simulink model "wlan_phy_rx_pmd"  (Simulink 7.8)
settings: Solver=VariableStepDiscrete  SolverMode=SingleTasking  StartTime=0.0  StopTime=simtime  RelTol=1e-3
PostLoadFcn:
  | wlan_phy_rx_init
InitFcn:
  | wlan_phy_rx_init
StartFcn:
  | wlan_phy_rx_init
values from neighbouring files (symbols this model uses):
  barker_seq20 = 0.95 * circshift(barker_seq20, [0 4]) ./ max(abs(barker_seq20))    [wlan_phy_rx_init.m]
  bit_scrambler_lfsr_bytes = bi2de(reshape(repmat(scr, 1, 8), 8, 127)', 'left-msb')    [wlan_phy_rx_init.m]
  deinterleave_ROM = [deinterleave_ROM interleave_64QAM zeros(1, 512-length(interleave_64QAM))]    [wlan_phy_rx_init.m]
  longCorr_coef_i = [3*real(LTS_corr)]    [wlan_phy_rx_init.m]
  longCorr_coef_q = [3*imag(LTS_corr)]    [wlan_phy_rx_init.m]
  MAX_CP_LEN = 32    [wlan_phy_rx_init.m]
  MAX_NCBPS = 288    [wlan_phy_rx_init.m]
  MAX_NUM_BYTES = 4096    [wlan_phy_rx_init.m]
  MAX_NUM_SC = 64    [wlan_phy_rx_init.m]
  MAX_NUM_SYMS = 600    [wlan_phy_rx_init.m]
  n_bits_preFFT_sampBuff = ceil(log2(4*MAX_NUM_SC))    [wlan_phy_rx_init.m]
  NCBPS_16QAM = 192    [wlan_phy_rx_init.m]
  NCBPS_64QAM = 288    [wlan_phy_rx_init.m]
  NCBPS_BPSK = 48    [wlan_phy_rx_init.m]
  NCBPS_QPSK = 96    [wlan_phy_rx_init.m]
  NDBPS_16QAM12 = 96    [wlan_phy_rx_init.m]
  NDBPS_16QAM34 = 144    [wlan_phy_rx_init.m]
  NDBPS_64QAM23 = 192    [wlan_phy_rx_init.m]
  NDBPS_64QAM34 = 216    [wlan_phy_rx_init.m]
  NDBPS_BPSK12 = 24    [wlan_phy_rx_init.m]
  NDBPS_BPSK34 = 36    [wlan_phy_rx_init.m]
  NDBPS_QPSK12 = 48    [wlan_phy_rx_init.m]
  NDBPS_QPSK34 = 72    [wlan_phy_rx_init.m]
  paylod_vec_samp_time = 8    [wlan_phy_rx_init.m]
  PHY_MIN_PKT_LEN = 14    [wlan_phy_rx_init.m]
  scr_ind_rev = zeros(1,128)    [wlan_phy_rx_init.m]
undefined symbols (not defined in the file or in its folder): gcbh, xlBlockLoadCallback, xlGetNormalizedPeriod, xlipgetpartsetting
libraries: xbsIndex_r4 x3119, simulink x1, xbsEDKLib_r4 x1
(part 3 of 19)

== system wlan_phy_rx_pmd/FCS & Pkt Buf ==
  DSSS data : Inport
  OFDM data : Inport  Port=2
  Bus Selector : BusSelector  OutputSignals=byte_valid,byte_index,byte,last_byte
  Bus Selector1 : BusSelector  OutputSignals=byte_valid,byte_index,byte,last_byte
  Bytes In : Scope  NumInputPorts=8
      settings: TimeRange=10000  YMin=0~0~0~-1~-1~-1~-1~-1  YMax=1~90~225~1~1~1~1~1  SaveName=ScopeData36
  FCS Calc : Subsystem
  Gateway Out{1..4,8..9,11..12} (8 alike) : xbsIndex_r4/Gateway Out  inherit_from_input=off  hdl_port=off  timing_constraint=None  locs_specified=off  LOCs={}  UseAsDAC=off  DACChannel='1'  block_type=gatewayout
  Pkt Buf Interface : Subsystem
  wires:
  OFDM data/1 -> Bus Selector/1, Pkt Buf Interface/2(OFDM data), FCS Calc/2(OFDM data)
  DSSS data/1 -> Bus Selector1/1, Pkt Buf Interface/1(DSSS data), FCS Calc/1(DSSS data)
  Bus Selector1/1 -> Gateway Out9/1  "<byte_valid>"
  Bus Selector1/2 -> Gateway Out8/1  "<byte_index>"
  Bus Selector1/3 -> Gateway Out11/1  "<byte>"
  Bus Selector1/4 -> Gateway Out12/1  "<last_byte>"
  Bus Selector/1 -> Gateway Out1/1  "<byte_valid>"
  Bus Selector/2 -> Gateway Out2/1  "<byte_index>"
  Bus Selector/3 -> Gateway Out3/1  "<byte>"
  Bus Selector/4 -> Gateway Out4/1  "<last_byte>"
  Gateway Out9/1 -> Bytes In/1  "DSSS Valid"
  Gateway Out8/1 -> Bytes In/2  "DSSS Byte Index"
  Gateway Out11/1 -> Bytes In/3  "DSSS Byte"
  Gateway Out12/1 -> Bytes In/4  "DSSS Last Byte"
  Gateway Out1/1 -> Bytes In/5  "OFDM Valid"
  Gateway Out2/1 -> Bytes In/6  "OFDM Byte Index"
  Gateway Out3/1 -> Bytes In/7  "OFDM Byte"
  Gateway Out4/1 -> Bytes In/8  "OFDM Last Byte"

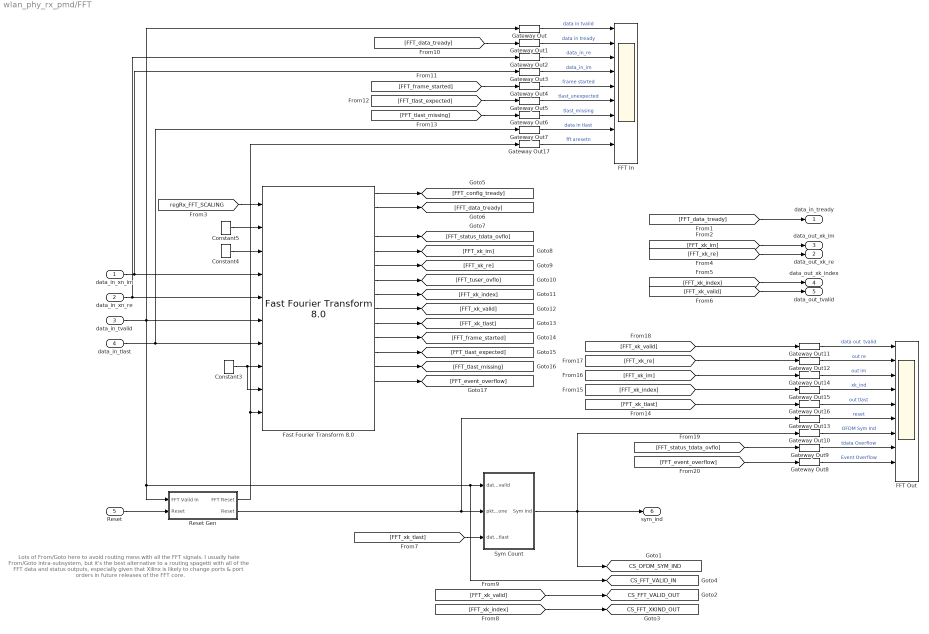
== system wlan_phy_rx_pmd/FFT ==
  note: Lots of From/Goto here to avoid routing
      | mess with all the FFT signals. I usually hate
      | From/Goto intra-subsystem, but it's the best
      | alternative to a routing spagetti with all of the
      | FFT data and status outputs, especially given
      | that Xilinx is likely to change ports & port orders
      | in future releases of the FFT core.
  data_in_xn_im : Inport
  data_in_xn_re : Inport  Port=2
  data_in_tvalid : Inport  Port=3
  data_in_tlast : Inport  Port=4
  Reset : Inport  Port=5
  Constant3 : xbsIndex_r4/Constant  const=1  gui_display_data_type=Boolean  arith_type=Boolean  n_bits=1  bin_pt=0  preci_type=Single  exp_width=8  frac_width=24  explicit_period=on  period=1  equ=P=C  opselect=C  inp2=PCIN>>17  opr=+  inp1=P  carry=CIN  dbl_ovrd=off  block_type=constant
  Constant4 : xbsIndex_r4/Constant  const=1  gui_display_data_type=Boolean  arith_type=Boolean  n_bits=1  bin_pt=0  preci_type=Single  exp_width=8  frac_width=24  explicit_period=on  period=1  equ=P=C  opselect=C  inp2=PCIN>>17  opr=+  inp1=P  carry=CIN  dbl_ovrd=off  block_type=constant
  Constant5 : xbsIndex_r4/Constant  const=1  gui_display_data_type=Fixed-point  arith_type=Unsigned  n_bits=1  bin_pt=0  preci_type=Single  exp_width=8  frac_width=24  explicit_period=on  period=1  equ=P=C  opselect=C  inp2=PCIN>>17  opr=+  inp1=P  carry=CIN  dbl_ovrd=off  block_type=constant
  FFT In : Scope  NumInputPorts=10
      settings: TimeRange=5000  YMin=0~0~-0.2~-1~0~-1~0~-1~-5~-5  YMax=1~1~0.8~1~1~1~1~1~5~5
  FFT Out : Scope  NumInputPorts=10
      settings: TimeRange=5000  YMin=0~-0.4~-0.4~0~0~-1~0~-1~-5~-5  YMax=1~0.4~0.4~70~1~1~1~1~5~5  SaveName=ScopeData29
  "Fast Fourier Transform 8.0 " : xbsIndex_r4/Fast Fourier Transform 8.0   transform_length=64  target_clock_frequency=160  target_data_throughput=50  implementation_options=radix_4_burst_io  run_time_configurable_transform_length=off  phase_factor_width=16  scaling_options=scaled  rounding_modes=truncation  aclken=off  aresetn=on  cyclic_prefix_insertion=off  output_ordering=natural_order  throttle_scheme=nonrealtime  xk_index=on  ovflo=on  trim_axipin_name=on  memory_options_data=block_ram  memory_options_phase_factors=block_ram  number_of_stages_using_block_ram_for_data_and_phase_factors=0  memory_options_reorder=block_ram  memory_options_hybrid=off  complex_mult_type=use_mults_performance  butterfly_type=use_luts  channels=1  input_ordering=natural_order  input_width=16  ip_name=Fast Fourier Transform  ip_version=8.0  dsptool_ready=true  ipcore_usecache=true  ipcore_useipmodelcache=true  wrapper_available=true  port_translation_map={ 'aclken' => 'en', 'aresetn' => 'rst'}  block_type=xfft_v8_0
  Gateway Out{,1..17} (18 alike) : xbsIndex_r4/Gateway Out  inherit_from_input=off  hdl_port=off  timing_constraint=None  locs_specified=off  LOCs={}  UseAsDAC=off  DACChannel='1'  block_type=gatewayout
  Reset Gen : Subsystem
  Sym Count : Subsystem
  data_in_tready : Outport
  data_out_xk_re : Outport  Port=2
  data_out_xk_im : Outport  Port=3
  data_out_xk_index : Outport  Port=4
  data_out_tvalid : Outport  Port=5
  sym_ind : Outport  Port=6
  tag scope: CS_FFT_VALID_IN=global, CS_FFT_VALID_OUT=global, CS_FFT_XKIND_OUT=global, CS_OFDM_SYM_IND=global
  wires:
  Gateway Out13/1 -> FFT Out/6  "reset"
  Gateway Out16/1 -> FFT Out/5  "out tlast"
  Gateway Out15/1 -> FFT Out/4  "xk_ind"
  Gateway Out14/1 -> FFT Out/3  "out im"
  Gateway Out12/1 -> FFT Out/2  "out re"
  Gateway Out11/1 -> FFT Out/1  "data out  tvalid"
  [FFT_xk_index] -> data_out_xk_index/1
  [FFT_xk_re] -> data_out_xk_re/1
  [FFT_xk_tlast] -> Sym Count/3(data_out_tlast)
  [FFT_xk_valid] -> data_out_tvalid/1
  [FFT_xk_im] -> data_out_xk_im/1
  [FFT_data_tready] -> data_in_tready/1
  Gateway Out7/1 -> FFT In/8  "data in tlast"
  Gateway Out6/1 -> FFT In/7  "tlast_missing"
  Gateway Out5/1 -> FFT In/6  "tlast_unexpected"
  Gateway Out4/1 -> FFT In/5  "frame started"
  Gateway Out3/1 -> FFT In/4  "data_in_im"
  Gateway Out2/1 -> FFT In/3  "data_in_re"
  Gateway Out1/1 -> FFT In/2  "data in tready"
  Gateway Out/1 -> FFT In/1  "data in tvalid"
  "Fast Fourier Transform 8.0 "/1 -> [FFT_config_tready]
  "Fast Fourier Transform 8.0 "/2 -> [FFT_data_tready]
  "Fast Fourier Transform 8.0 "/4 -> [FFT_status_tdata_ovflo]
  "Fast Fourier Transform 8.0 "/5 -> [FFT_xk_im]
  "Fast Fourier Transform 8.0 "/6 -> [FFT_xk_re]
  "Fast Fourier Transform 8.0 "/7 -> [FFT_tuser_ovflo]
  "Fast Fourier Transform 8.0 "/8 -> [FFT_xk_index]
  "Fast Fourier Transform 8.0 "/9 -> [FFT_xk_valid]
  "Fast Fourier Transform 8.0 "/10 -> [FFT_xk_tlast]
  "Fast Fourier Transform 8.0 "/11 -> [FFT_frame_started]
  "Fast Fourier Transform 8.0 "/12 -> [FFT_tlast_expected]
  "Fast Fourier Transform 8.0 "/13 -> [FFT_tlast_missing]
  "Fast Fourier Transform 8.0 "/14 -> [FFT_event_overflow]
  Constant3/1 -> "Fast Fourier Transform 8.0 "/8, "Fast Fourier Transform 8.0 "/9
  Constant4/1 -> "Fast Fourier Transform 8.0 "/3
  Constant5/1 -> "Fast Fourier Transform 8.0 "/2
  [regRx_FFT_SCALING] -> "Fast Fourier Transform 8.0 "/1
  [FFT_xk_index] -> [CS_FFT_XKIND_OUT]
  [FFT_xk_valid] -> [CS_FFT_VALID_OUT]
  Reset Gen/2(" Reset") -> Sym Count/2(pkt_done), Gateway Out13/1
  Reset/1 -> Reset Gen/2(Reset)
  data_in_tvalid/1 -> "Fast Fourier Transform 8.0 "/6, Reset Gen/1(FFT Valid In), Sym Count/1(data_in_tvalid), [CS_FFT_VALID_IN], Gateway Out/1
  Sym Count/1(Sym Ind) -> sym_ind/1, Gateway Out10/1, [CS_OFDM_SYM_IND]
  data_in_tlast/1 -> "Fast Fourier Transform 8.0 "/7, Gateway Out7/1
  data_in_xn_im/1 -> "Fast Fourier Transform 8.0 "/4, Gateway Out3/1
  data_in_xn_re/1 -> "Fast Fourier Transform 8.0 "/5, Gateway Out2/1
  Gateway Out10/1 -> FFT Out/7  "OFDM Sym Ind"
  Gateway Out9/1 -> FFT Out/8  "tdata Overflow"
  [FFT_data_tready] -> Gateway Out1/1
  [FFT_frame_started] -> Gateway Out4/1
  [FFT_tlast_expected] -> Gateway Out5/1
  [FFT_tlast_missing] -> Gateway Out6/1
  [FFT_xk_tlast] -> Gateway Out16/1
  [FFT_xk_index] -> Gateway Out15/1
  [FFT_xk_im] -> Gateway Out14/1
  [FFT_xk_re] -> Gateway Out12/1
  [FFT_xk_valid] -> Gateway Out11/1
  [FFT_status_tdata_ovflo] -> Gateway Out9/1
  [FFT_event_overflow] -> Gateway Out8/1
  Gateway Out8/1 -> FFT Out/9  "Event Overflow"
  Reset Gen/1(FFT Reset) -> "Fast Fourier Transform 8.0 "/10, Gateway Out17/1
  Gateway Out17/1 -> FFT In/9  "fft aresetn"

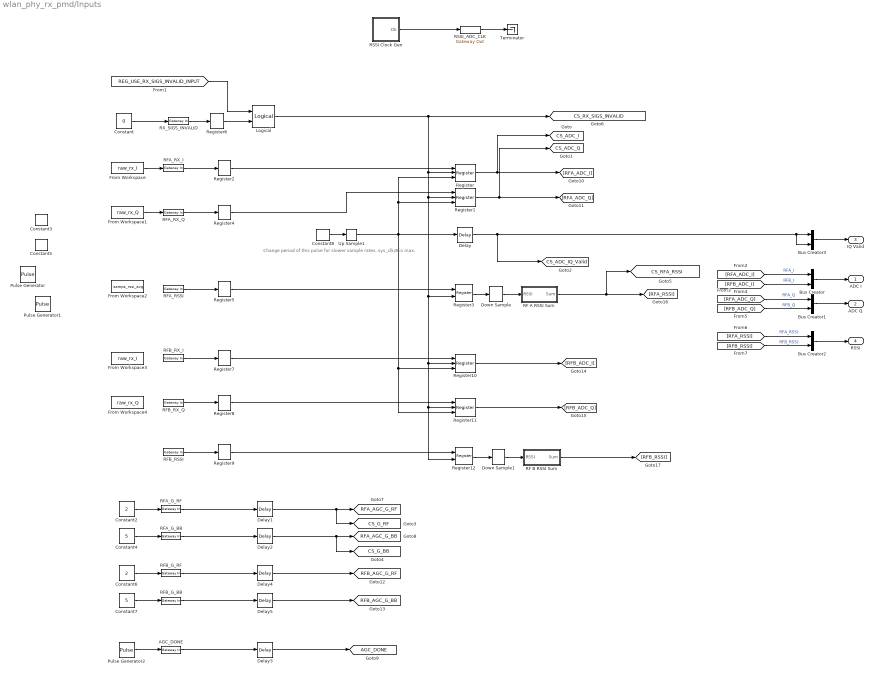
== system wlan_phy_rx_pmd/Inputs ==
  note: Change period of this pulse for slower
      | sample rates. sys_clk/8 is max.
  AGC_DONE : xbsIndex_r4/Gateway In  gui_display_data_type=Boolean  arith_type=Boolean  n_bits=5  bin_pt=0  preci_type=Single  exp_width=8  frac_width=24  quantization="Round  (unbiased: +/- Inf)"  overflow=Saturate  period=1  dbl_ovrd=off  timing_constraint=None  locs_specified=off  LOCs={}  UseAsADC=off  ADCChannel='1'  inherit_from_input=off  hdl_port=on  block_type=gatewayin
  Bus Creator{,1..3} (4 alike) : BusCreator  Inputs=2
  Constant : Constant  Value=0
  Constant2 : Constant  Value=2
  Constant3 : xbsIndex_r4/Constant  const=0  gui_display_data_type=Fixed-point  arith_type=Signed (2's comp)  n_bits=16  bin_pt=15  preci_type=Single  exp_width=8  frac_width=24  explicit_period=on  period=1  equ=P=C  opselect=C  inp2=PCIN>>17  opr=+  inp1=P  carry=CIN  dbl_ovrd=off  block_type=constant
  Constant4 : Constant  Value=5
  Constant5 : xbsIndex_r4/Constant  const=0  gui_display_data_type=Fixed-point  arith_type=Signed (2's comp)  n_bits=16  bin_pt=15  preci_type=Single  exp_width=8  frac_width=24  explicit_period=on  period=1  equ=P=C  opselect=C  inp2=PCIN>>17  opr=+  inp1=P  carry=CIN  dbl_ovrd=off  block_type=constant
  Constant6 : Constant  Value=2
  Constant7 : Constant  Value=5
  Constant8 : xbsIndex_r4/Constant  const=1  gui_display_data_type=Boolean  arith_type=Boolean  n_bits=16  bin_pt=14  preci_type=Single  exp_width=8  frac_width=24  explicit_period=on  period=8  equ=P=C  opselect=C  inp2=PCIN>>17  opr=+  inp1=P  carry=CIN  dbl_ovrd=off  block_type=constant
  Delay{,1..5} (6 alike) : xbsIndex_r4/Delay  rst=off  infoeditControl=Selection of Reset will increase slice count due to use of real FFs and instead of SRLs  en=off  latency=1  dbl_ovrd=off  reg_retiming=off  block_type=delay
  Down Sample : xbsIndex_r4/Down Sample  sample_ratio=16  sample_phase="Last Value of Frame  (most efficient)"  en=off  latency=1  dbl_ovrd=off  block_type=dsamp
  Down Sample1 : xbsIndex_r4/Down Sample  sample_ratio=16  sample_phase="Last Value of Frame  (most efficient)"  en=off  latency=1  dbl_ovrd=off  block_type=dsamp
  From Workspace : FromWorkspace  VariableName=raw_rx_I  SampleTime=paylod_vec_samp_time  Interpolate=off  OutputAfterFinalValue=Setting to zero
  From Workspace1 : FromWorkspace  VariableName=raw_rx_Q  SampleTime=paylod_vec_samp_time  Interpolate=off  OutputAfterFinalValue=Setting to zero
  From Workspace2 : FromWorkspace  VariableName=samps_rssi_avg  SampleTime=paylod_vec_samp_time  Interpolate=off  OutputAfterFinalValue=Setting to zero
  From Workspace3 : FromWorkspace  VariableName=raw_rx_I  SampleTime=paylod_vec_samp_time  Interpolate=off  OutputAfterFinalValue=Setting to zero
  From Workspace4 : FromWorkspace  VariableName=raw_rx_Q  SampleTime=paylod_vec_samp_time  Interpolate=off  OutputAfterFinalValue=Setting to zero
  Logical : xbsIndex_r4/Logical  logical_function=AND  inputs=2  en=off  latency=0  precision=Full  arith_type=Unsigned  n_bits=16  bin_pt=0  align_bp=on  dbl_ovrd=off  block_type=logical
  Pulse Generator : DiscretePulseGenerator  Period=8
  Pulse Generator1 : DiscretePulseGenerator  Amplitude=800  Period=1e6  PulseWidth=1e4
  Pulse Generator2 : DiscretePulseGenerator  Period=1e7  PhaseDelay=930
  RF A RSSI Sum : Subsystem
  RF B RSSI Sum : Subsystem
  RFA_G_BB : xbsIndex_r4/Gateway In  gui_display_data_type=Fixed-point  arith_type=Unsigned  n_bits=5  bin_pt=0  preci_type=Single  exp_width=8  frac_width=24  quantization="Round  (unbiased: +/- Inf)"  overflow=Saturate  period=1  dbl_ovrd=off  timing_constraint=None  locs_specified=off  LOCs={}  UseAsADC=off  ADCChannel='1'  inherit_from_input=off  hdl_port=on  block_type=gatewayin
  RFA_G_RF : xbsIndex_r4/Gateway In  gui_display_data_type=Fixed-point  arith_type=Unsigned  n_bits=2  bin_pt=0  preci_type=Single  exp_width=8  frac_width=24  quantization="Round  (unbiased: +/- Inf)"  overflow=Saturate  period=1  dbl_ovrd=off  timing_constraint=None  locs_specified=off  LOCs={}  UseAsADC=off  ADCChannel='1'  inherit_from_input=off  hdl_port=on  block_type=gatewayin
  RFA_RSSI : xbsIndex_r4/Gateway In  gui_display_data_type=Fixed-point  arith_type=Unsigned  n_bits=10  bin_pt=0  preci_type=Single  exp_width=8  frac_width=24  quantization="Round  (unbiased: +/- Inf)"  overflow=Saturate  period=1  dbl_ovrd=off  timing_constraint=None  locs_specified=off  LOCs={}  UseAsADC=off  ADCChannel='1'  inherit_from_input=off  hdl_port=on  block_type=gatewayin
  RFA_RX_I : xbsIndex_r4/Gateway In  gui_display_data_type=Fixed-point  arith_type="Signed  (2's comp)"  n_bits=16  bin_pt=15  preci_type=Single  exp_width=8  frac_width=24  quantization="Round  (unbiased: +/- Inf)"  overflow=Saturate  period=1  dbl_ovrd=off  timing_constraint=None  locs_specified=off  LOCs={}  UseAsADC=off  ADCChannel='1'  inherit_from_input=off  hdl_port=on  block_type=gatewayin
  RFA_RX_Q : xbsIndex_r4/Gateway In  gui_display_data_type=Fixed-point  arith_type="Signed  (2's comp)"  n_bits=16  bin_pt=15  preci_type=Single  exp_width=8  frac_width=24  quantization="Round  (unbiased: +/- Inf)"  overflow=Saturate  period=1  dbl_ovrd=off  timing_constraint=None  locs_specified=off  LOCs={}  UseAsADC=off  ADCChannel='1'  inherit_from_input=off  hdl_port=on  block_type=gatewayin
  RFB_G_BB : xbsIndex_r4/Gateway In  gui_display_data_type=Fixed-point  arith_type=Unsigned  n_bits=5  bin_pt=0  preci_type=Single  exp_width=8  frac_width=24  quantization="Round  (unbiased: +/- Inf)"  overflow=Saturate  period=1  dbl_ovrd=off  timing_constraint=None  locs_specified=off  LOCs={}  UseAsADC=off  ADCChannel='1'  inherit_from_input=off  hdl_port=on  block_type=gatewayin
  RFB_G_RF : xbsIndex_r4/Gateway In  gui_display_data_type=Fixed-point  arith_type=Unsigned  n_bits=2  bin_pt=0  preci_type=Single  exp_width=8  frac_width=24  quantization="Round  (unbiased: +/- Inf)"  overflow=Saturate  period=1  dbl_ovrd=off  timing_constraint=None  locs_specified=off  LOCs={}  UseAsADC=off  ADCChannel='1'  inherit_from_input=off  hdl_port=on  block_type=gatewayin
  RFB_RSSI : xbsIndex_r4/Gateway In  gui_display_data_type=Fixed-point  arith_type=Unsigned  n_bits=10  bin_pt=0  preci_type=Single  exp_width=8  frac_width=24  quantization="Round  (unbiased: +/- Inf)"  overflow=Saturate  period=1  dbl_ovrd=off  timing_constraint=None  locs_specified=off  LOCs={}  UseAsADC=off  ADCChannel='1'  inherit_from_input=off  hdl_port=on  block_type=gatewayin
  RFB_RX_I : xbsIndex_r4/Gateway In  gui_display_data_type=Fixed-point  arith_type="Signed  (2's comp)"  n_bits=16  bin_pt=15  preci_type=Single  exp_width=8  frac_width=24  quantization="Round  (unbiased: +/- Inf)"  overflow=Saturate  period=1  dbl_ovrd=off  timing_constraint=None  locs_specified=off  LOCs={}  UseAsADC=off  ADCChannel='1'  inherit_from_input=off  hdl_port=on  block_type=gatewayin
  RFB_RX_Q : xbsIndex_r4/Gateway In  gui_display_data_type=Fixed-point  arith_type="Signed  (2's comp)"  n_bits=16  bin_pt=15  preci_type=Single  exp_width=8  frac_width=24  quantization="Round  (unbiased: +/- Inf)"  overflow=Saturate  period=1  dbl_ovrd=off  timing_constraint=None  locs_specified=off  LOCs={}  UseAsADC=off  ADCChannel='1'  inherit_from_input=off  hdl_port=on  block_type=gatewayin
  RSSI Clock Gen : Subsystem
  RSSI_ADC_CLK : xbsIndex_r4/Gateway Out  inherit_from_input=off  hdl_port=on  timing_constraint=None  locs_specified=off  LOCs={}  UseAsDAC=off  DACChannel='1'  block_type=gatewayout
      settings: infoedit="Gateway out block.  Converts Xilinx fixed point inputs into ouputs of type Simulink integer, double, or fixed point.<P><P>Hardware notes:  In hardware these blocks become top level output ports or are discarded, depending on how they are configured."  block_version=8.2
  RX_SIGS_INVALID : xbsIndex_r4/Gateway In  gui_display_data_type=Boolean  arith_type=Boolean  n_bits=1  bin_pt=0  preci_type=Single  exp_width=8  frac_width=24  quantization="Round  (unbiased: +/- Inf)"  overflow=Saturate  period=1  dbl_ovrd=off  timing_constraint=None  locs_specified=off  LOCs={}  UseAsADC=off  ADCChannel='1'  inherit_from_input=off  hdl_port=on  block_type=gatewayin
  Register{,1,10..11} (4 alike) : xbsIndex_r4/Register  init=0  rst=on  en=on  dbl_ovrd=off  block_type=register
  Register12 : xbsIndex_r4/Register  init=0  rst=on  en=off  dbl_ovrd=off  block_type=register
  Register{2,4..9} (7 alike) : xbsIndex_r4/Register  init=0  rst=off  en=off  dbl_ovrd=off  block_type=register
  Register3 : xbsIndex_r4/Register  init=0  rst=on  en=off  dbl_ovrd=off  block_type=register
  Terminator : Terminator
  Up Sample1 : xbsIndex_r4/Up Sample  sample_ratio=8  copy_samples=off  en=off  latency=0  dbl_ovrd=off  block_type=usamp
  ADC I : Outport
  ADC Q : Outport  Port=2
  IQ Valid : Outport  Port=3
  RSSI : Outport  Port=4
  tag scope: AGC_DONE=global, CS_ADC_I=global, CS_ADC_IQ_Valid=global, CS_ADC_Q=global, CS_G_BB=global, CS_G_RF=global, CS_RFA_RSSI=global, CS_RX_SIGS_INVALID=global, RFA_AGC_G_BB=global, RFA_AGC_G_RF=global, RFB_AGC_G_BB=global, RFB_AGC_G_RF=global
  wires:
  From Workspace/1 -> RFA_RX_I/1
  From Workspace1/1 -> RFA_RX_Q/1
  RFA_RX_I/1 -> Register2/1
  RFA_RX_Q/1 -> Register4/1
  Register/1 -> [CS_ADC_I], [RFA_ADC_I]
  Register1/1 -> [CS_ADC_Q], [RFA_ADC_Q]
  Delay/1 -> [CS_ADC_IQ_Valid], Bus Creator3/1, Bus Creator3/2
  Register3/1 -> Down Sample/1
  Down Sample/1 -> RF A RSSI Sum/1(RSSI)
  RSSI_ADC_CLK/1 -> Terminator/1
  RSSI Clock Gen/1(Clk) -> RSSI_ADC_CLK/1
  RF A RSSI Sum/1(Sum) -> [CS_RFA_RSSI], [RFA_RSSI]
  Constant/1 -> RX_SIGS_INVALID/1
  RX_SIGS_INVALID/1 -> Register6/1
  Logical/1 -> Register/2, Register1/2, Register3/2, Register10/2, Register11/2, Register12/2, [CS_RX_SIGS_INVALID]
  Register6/1 -> Logical/2
  [REG_USE_RX_SIGS_INVALID_INPUT] -> Logical/1
  RFA_RSSI/1 -> Register5/1
  Register2/1 -> Register/1
  Register4/1 -> Register1/1
  Register5/1 -> Register3/1
  RFB_RX_I/1 -> Register7/1
  RFB_RX_Q/1 -> Register8/1
  RFB_RSSI/1 -> Register9/1
  Register9/1 -> Register12/1
  Register7/1 -> Register10/1
  Register8/1 -> Register11/1
  Register12/1 -> Down Sample1/1
  Down Sample1/1 -> RF B RSSI Sum/1(RSSI)
  Bus Creator/1 -> ADC I/1
  Bus Creator1/1 -> ADC Q/1
  Bus Creator2/1 -> RSSI/1
  Register10/1 -> [RFB_ADC_I]
  Register11/1 -> [RFB_ADC_Q]
  RF B RSSI Sum/1(Sum) -> [RFB_RSSI]
  [RFA_ADC_I] -> Bus Creator/1  "RFA_I"
  [RFB_ADC_I] -> Bus Creator/2  "RFB_I"
  [RFA_ADC_Q] -> Bus Creator1/1  "RFA_Q"
  [RFB_ADC_Q] -> Bus Creator1/2  "RFB_Q"
  [RFA_RSSI] -> Bus Creator2/1  "RFA_RSSI"
  [RFB_RSSI] -> Bus Creator2/2  "RFB_RSSI"
  RFA_G_RF/1 -> Delay1/1
  RFA_G_BB/1 -> Delay2/1
  Delay1/1 -> [CS_G_RF], [RFA_AGC_G_RF]
  Delay2/1 -> [CS_G_BB], [RFA_AGC_G_BB]
  AGC_DONE/1 -> Delay3/1
  Delay3/1 -> [AGC_DONE]
  RFB_G_RF/1 -> Delay4/1
  RFB_G_BB/1 -> Delay5/1
  Delay4/1 -> [RFB_AGC_G_RF]
  Delay5/1 -> [RFB_AGC_G_BB]
  Bus Creator3/1 -> IQ Valid/1
  Constant2/1 -> RFA_G_RF/1
  Constant4/1 -> RFA_G_BB/1
  Constant6/1 -> RFB_G_RF/1
  Constant7/1 -> RFB_G_BB/1
  Pulse Generator2/1 -> AGC_DONE/1
  Up Sample1/1 -> Delay/1, Register1/3, Register/3, Register10/3, Register11/3
  Constant8/1 -> Up Sample1/1

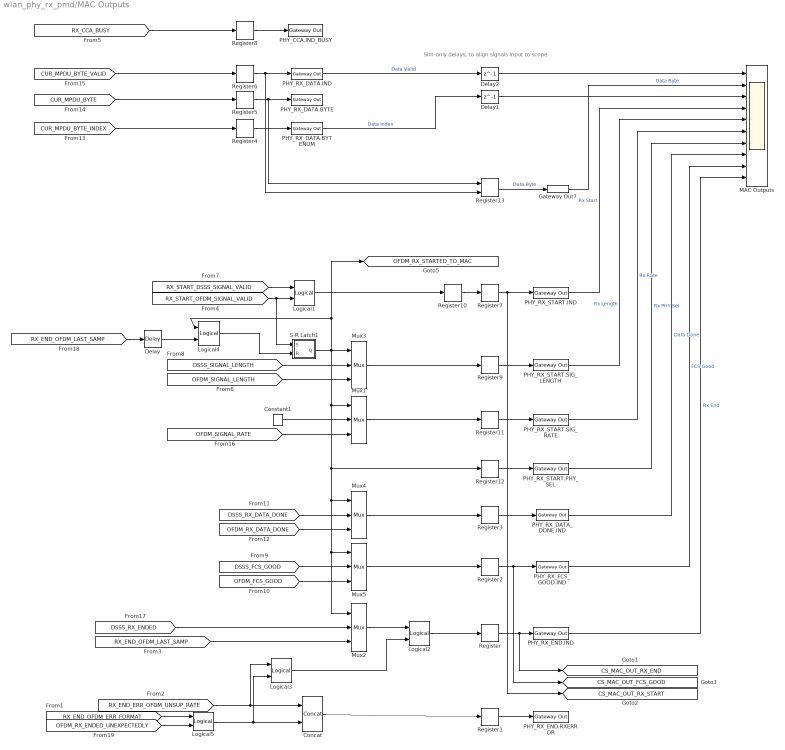
== system wlan_phy_rx_pmd/MAC Outputs ==
  note: Sim-only delays, to align signals
      | input to scope
  Concat : xbsIndex_r4/Concat  num_inputs=2  dbl_ovrd=off  block_type=concat
  Constant1 : xbsIndex_r4/Constant  const=1  gui_display_data_type=Fixed-point  arith_type=Unsigned  n_bits=4  bin_pt=0  preci_type=Single  exp_width=8  frac_width=24  explicit_period=off  period=1  equ=P=C  opselect=C  inp2=PCIN>>17  opr=+  inp1=P  carry=CIN  dbl_ovrd=off  block_type=constant
      settings: block_version=VER_STRING_GOES_HERE
  Delay : xbsIndex_r4/Delay  rst=off  infoeditControl=Selection of Reset will increase slice count due to use of real FFs and instead of SRLs  en=off  latency=8  dbl_ovrd=off  reg_retiming=off  block_type=delay
  Delay1 : Delay  DelayLength=1  SampleTime=1
  Delay2 : Delay  DelayLength=1  SampleTime=1
  Gateway Out7 : xbsIndex_r4/Gateway Out  inherit_from_input=off  hdl_port=off  timing_constraint=None  locs_specified=off  LOCs={}  UseAsDAC=off  DACChannel='1'  block_type=gatewayout
  Logical{1..3,5} (4 alike) : xbsIndex_r4/Logical  logical_function=OR  inputs=2  en=off  latency=0  precision=Full  arith_type=Unsigned  n_bits=16  bin_pt=0  align_bp=on  dbl_ovrd=off  block_type=logical
  Logical4 : xbsIndex_r4/Logical  logical_function=AND  inputs=2  en=off  latency=0  precision=Full  arith_type=Unsigned  n_bits=16  bin_pt=0  align_bp=on  dbl_ovrd=off  block_type=logical
  MAC Outputs : Scope  NumInputPorts=10
      settings: TimeRange=9204  YMin=-1~209~-1~-1~133~1~-1~-1~-1~-1  YMax=1~209~1~1~133~1~1~1~1~1  SaveName=ScopeData37
  Mux{1..5} (5 alike) : xbsIndex_r4/Mux  inputs=2  en=off  latency=0  precision=Full  arith_type=Unsigned  n_bits=16  bin_pt=14  quantization=Truncate  overflow=Wrap  dbl_ovrd=off  block_type=mux
  PHY_CCA.IND_BUSY : xbsIndex_r4/Gateway Out  inherit_from_input=off  hdl_port=on  timing_constraint=None  locs_specified=off  LOCs={}  UseAsDAC=off  DACChannel='1'  block_type=gatewayout
  PHY_RX_DATA.BYTE : xbsIndex_r4/Gateway Out  inherit_from_input=off  hdl_port=on  timing_constraint=None  locs_specified=off  LOCs={}  UseAsDAC=off  DACChannel='1'  block_type=gatewayout
  PHY_RX_DATA.BYTENUM : xbsIndex_r4/Gateway Out  inherit_from_input=off  hdl_port=on  timing_constraint=None  locs_specified=off  LOCs={}  UseAsDAC=off  DACChannel='1'  block_type=gatewayout
  PHY_RX_DATA.IND : xbsIndex_r4/Gateway Out  inherit_from_input=off  hdl_port=on  timing_constraint=None  locs_specified=off  LOCs={}  UseAsDAC=off  DACChannel='1'  block_type=gatewayout
  PHY_RX_DATA_DONE.IND : xbsIndex_r4/Gateway Out  inherit_from_input=off  hdl_port=on  timing_constraint=None  locs_specified=off  LOCs={}  UseAsDAC=off  DACChannel='1'  block_type=gatewayout
  PHY_RX_END.IND : xbsIndex_r4/Gateway Out  inherit_from_input=off  hdl_port=on  timing_constraint=None  locs_specified=off  LOCs={}  UseAsDAC=off  DACChannel='1'  block_type=gatewayout
  PHY_RX_END.RXERROR : xbsIndex_r4/Gateway Out  inherit_from_input=off  hdl_port=on  timing_constraint=None  locs_specified=off  LOCs={}  UseAsDAC=off  DACChannel='1'  block_type=gatewayout
  PHY_RX_FCS_GOOD.IND : xbsIndex_r4/Gateway Out  inherit_from_input=off  hdl_port=on  timing_constraint=None  locs_specified=off  LOCs={}  UseAsDAC=off  DACChannel='1'  block_type=gatewayout
  PHY_RX_START.IND : xbsIndex_r4/Gateway Out  inherit_from_input=off  hdl_port=on  timing_constraint=None  locs_specified=off  LOCs={}  UseAsDAC=off  DACChannel='1'  block_type=gatewayout
  PHY_RX_START.PHY_SEL : xbsIndex_r4/Gateway Out  inherit_from_input=off  hdl_port=on  timing_constraint=None  locs_specified=off  LOCs={}  UseAsDAC=off  DACChannel='1'  block_type=gatewayout
  PHY_RX_START.SIG_LENGTH : xbsIndex_r4/Gateway Out  inherit_from_input=off  hdl_port=on  timing_constraint=None  locs_specified=off  LOCs={}  UseAsDAC=off  DACChannel='1'  block_type=gatewayout
  PHY_RX_START.SIG_RATE : xbsIndex_r4/Gateway Out  inherit_from_input=off  hdl_port=on  timing_constraint=None  locs_specified=off  LOCs={}  UseAsDAC=off  DACChannel='1'  block_type=gatewayout
  Register{,1..12} (13 alike) : xbsIndex_r4/Register  init=0  rst=off  en=off  dbl_ovrd=off  block_type=register
  Register13 : xbsIndex_r4/Register  init=0  rst=off  en=on  dbl_ovrd=off  block_type=register
  S-R Latch1 : Subsystem
  tag scope: CS_MAC_OUT_FCS_GOOD=global, CS_MAC_OUT_RX_END=global, CS_MAC_OUT_RX_START=global, OFDM_RX_STARTED_TO_MAC=global
  wires:
  Register/1 -> PHY_RX_END.IND/1, [CS_MAC_OUT_RX_END]
  Register1/1 -> PHY_RX_END.RXERROR/1
  Register2/1 -> PHY_RX_FCS_GOOD.IND/1, [CS_MAC_OUT_FCS_GOOD]
  Register3/1 -> PHY_RX_DATA_DONE.IND/1
  [CUR_MPDU_BYTE] -> Register5/1
  [CUR_MPDU_BYTE_VALID] -> Register6/1
  [CUR_MPDU_BYTE_INDEX] -> Register4/1
  Register4/1 -> PHY_RX_DATA.BYTENUM/1
  Register5/1 -> PHY_RX_DATA.BYTE/1, Register13/1
  Register6/1 -> PHY_RX_DATA.IND/1, Register13/2
  [RX_CCA_BUSY] -> Register8/1
  Register7/1 -> PHY_RX_START.IND/1, [CS_MAC_OUT_RX_START]
  Register8/1 -> PHY_CCA.IND_BUSY/1
  Register9/1 -> PHY_RX_START.SIG_LENGTH/1
  [DSSS_FCS_GOOD] -> Mux5/2
  [OFDM_FCS_GOOD] -> Mux5/3
  [DSSS_RX_DATA_DONE] -> Mux4/2
  [OFDM_RX_DATA_DONE] -> Mux4/3
  Mux4/1 -> Register3/1
  [RX_START_DSSS_SIGNAL_VALID] -> Logical1/1
  [RX_START_OFDM_SIGNAL_VALID] -> Logical1/2, S-R Latch1/1(S)
  Logical1/1 -> Register10/1
  S-R Latch1/1(Q) -> Mux3/1, Mux1/1, Mux4/1, Mux2/1, Mux5/1, Register12/1, Logical4/1, [OFDM_RX_STARTED_TO_MAC]
  [OFDM_SIGNAL_LENGTH] -> Mux3/3
  [DSSS_SIGNAL_LENGTH] -> Mux3/2
  Mux3/1 -> Register9/1
  Register11/1 -> PHY_RX_START.SIG_RATE/1
  [OFDM_SIGNAL_RATE] -> Mux1/3
  Constant1/1 -> Mux1/2
  Mux1/1 -> Register11/1
  Logical4/1 -> S-R Latch1/2(R)
  [RX_END_OFDM_LAST_SAMP] -> Mux2/3
  Logical5/1 -> Concat/2, Logical3/2
  [RX_END_ERR_OFDM_UNSUP_RATE] -> Concat/1, Logical3/1
  Concat/1 -> Register1/1
  Mux2/1 -> Logical2/1
  [DSSS_RX_ENDED] -> Mux2/2
  Logical3/1 -> Logical2/2
  Logical2/1 -> Register/1
  PHY_RX_DATA.IND/1 -> Delay2/1  "Data Valid"
  PHY_RX_DATA.BYTENUM/1 -> Delay1/1  "Data Index"
  PHY_RX_START.IND/1 -> MAC Outputs/4  "Rx Start"
  PHY_RX_START.SIG_LENGTH/1 -> MAC Outputs/5  "Rx Length"
  Mux5/1 -> Register2/1
  [RX_END_OFDM_LAST_SAMP] -> Delay/1
  Register10/1 -> Register7/1
  PHY_RX_START.SIG_RATE/1 -> MAC Outputs/6  "Rx Rate"
  PHY_RX_START.PHY_SEL/1 -> MAC Outputs/7  "Rx PHY Sel"
  Register12/1 -> PHY_RX_START.PHY_SEL/1
  PHY_RX_DATA_DONE.IND/1 -> MAC Outputs/8  "Data Done"
  PHY_RX_FCS_GOOD.IND/1 -> MAC Outputs/9  "FCS Good"
  PHY_RX_END.IND/1 -> MAC Outputs/10  "Rx End"
  Delay/1 -> Logical4/2
  [RX_END_OFDM_ERR_FORMAT] -> Logical5/1
  [OFDM_RX_ENDED_UNEXPECTEDLY] -> Logical5/2
  Register13/1 -> Gateway Out7/1  "Data Byte"
  Gateway Out7/1 -> MAC Outputs/2  "Data Byte"
  Delay1/1 -> MAC Outputs/3
  Delay2/1 -> MAC Outputs/1
